simulink model "Scenario_4_power_system_modeling"  (R2023b)
settings: Solver=ode4  StartTime=0.0  StopTime=90  FixedStep=0.01  RelTol=1e-3
values from neighbouring files (symbols this model uses):
  a12 = -1    [simulation_3_Area_scenario.m]    (also in simulation_scenario_1_2_3.m)
  a13 = -1    [simulation_3_Area_scenario.m]
  a23 = -1    [simulation_3_Area_scenario.m]
  b = 0.05    [simulation_3_Area_scenario.m]
  B1 = (1/Rtotal_1) + D1    (= 20.9)    [simulation_3_Area_scenario.m]    (also in simulation_scenario_1_2_3.m = 40.9)
  B2 = (1/Rtotal_2) + D2    (= 21.5)    [simulation_3_Area_scenario.m]    (also in simulation_scenario_1_2_3.m = 41.5)
  B3 = (1/Rtotal_3) + D3    (= 31.3)    [simulation_3_Area_scenario.m]
  c = 1    [simulation_3_Area_scenario.m]
  D1 = 0.9    [simulation_3_Area_scenario.m]    (also in simulation_scenario_1_2_3.m)
  D2 = 1.5    [simulation_3_Area_scenario.m]    (also in simulation_scenario_1_2_3.m)
  D3 = 1.3    [simulation_3_Area_scenario.m]
  Fhp = 0.4    [simulation_3_Area_scenario.m]    (also in simulation_scenario_1_2_3.m)
  Fhp2 = 0.3    [simulation_3_Area_scenario.m]
  Fhp_3 = 0.35    [simulation_3_Area_scenario.m]
  H1 = H1_values(i)    [simulation_3_Area_scenario.m]    (also in simulation_scenario_1_2_3.m)
  H2 = H2_values(i)    [simulation_3_Area_scenario.m]    (also in simulation_scenario_1_2_3.m)
  H3 = H3_values(i)    [simulation_3_Area_scenario.m]
  KI1 = 0.25    [simulation_3_Area_scenario.m]    (also in simulation_scenario_1_2_3.m)
  KI2 = 0.25    [simulation_3_Area_scenario.m]    (also in simulation_scenario_1_2_3.m)
  KI3 = 0.25    [simulation_3_Area_scenario.m]    (also in simulation_scenario_1_2_3.m)
  KI4 = 0.25    [simulation_3_Area_scenario.m]    (also in simulation_scenario_1_2_3.m)
  KI5 = 0.25    [simulation_3_Area_scenario.m]
  KI6 = 0.25    [simulation_3_Area_scenario.m]
  KI7 = 0.25    [simulation_3_Area_scenario.m]
  R1 = 0.1    [simulation_3_Area_scenario.m]    (also in simulation_scenario_1_2_3.m = 0.05)
  R2 = 0.1    [simulation_3_Area_scenario.m]    (also in simulation_scenario_1_2_3.m = 0.05)
  R4 = 0.1    [simulation_3_Area_scenario.m]    (also in simulation_scenario_1_2_3.m = 0.05)
  R5 = 0.1    [simulation_3_Area_scenario.m]
  R6 = 0.1    [simulation_3_Area_scenario.m]
  R7 = 0.1    [simulation_3_Area_scenario.m]
  Rp = 0.05    [simulation_3_Area_scenario.m]    (also in simulation_scenario_1_2_3.m)
  Rt = 0.38    [simulation_3_Area_scenario.m]    (also in simulation_scenario_1_2_3.m)
  T12 = 2    [simulation_3_Area_scenario.m]    (also in simulation_scenario_1_2_3.m)
  T13 = 2    [simulation_3_Area_scenario.m]
  T23 = 2    [simulation_3_Area_scenario.m]
  Tcd = 0.2    [simulation_3_Area_scenario.m]
  Tch1 = 0.39    [simulation_3_Area_scenario.m]    (also in simulation_scenario_1_2_3.m)
  Tch2 = 0.5    [simulation_3_Area_scenario.m]    (also in simulation_scenario_1_2_3.m)
  Tch4 = 0.45    [simulation_3_Area_scenario.m]    (also in simulation_scenario_1_2_3.m)
  Tch5 = 0.4    [simulation_3_Area_scenario.m]
  Tch6 = 0.3    [simulation_3_Area_scenario.m]
  Tcr = 0.01    [simulation_3_Area_scenario.m]
  Tf = 0.23    [simulation_3_Area_scenario.m]
  Tg1 = 0.23    [simulation_3_Area_scenario.m]    (also in simulation_scenario_1_2_3.m)
  Tg2 = 0.14    [simulation_3_Area_scenario.m]    (also in simulation_scenario_1_2_3.m)
  Tg3 = 0.4    [simulation_3_Area_scenario.m]    (also in simulation_scenario_1_2_3.m)
  Tg4 = 0.3    [simulation_3_Area_scenario.m]    (also in simulation_scenario_1_2_3.m)
  Tg5 = 0.1    [simulation_3_Area_scenario.m]
  Tg6 = 0.08    [simulation_3_Area_scenario.m]
  Tr = 5    [simulation_3_Area_scenario.m]    (also in simulation_scenario_1_2_3.m)
  Trh = 7    [simulation_3_Area_scenario.m]    (also in simulation_scenario_1_2_3.m)
  Trh2 = 10    [simulation_3_Area_scenario.m]
  Trh_3 = 5    [simulation_3_Area_scenario.m]
  Tw = 2.25    [simulation_3_Area_scenario.m]    (also in simulation_scenario_1_2_3.m)
  X = 0.6    [simulation_3_Area_scenario.m]
  Y = 1    [simulation_3_Area_scenario.m]
libraries: simulink x4, dspfdesign x3
settings Model: Creator=themi  LastModifiedBy=themi

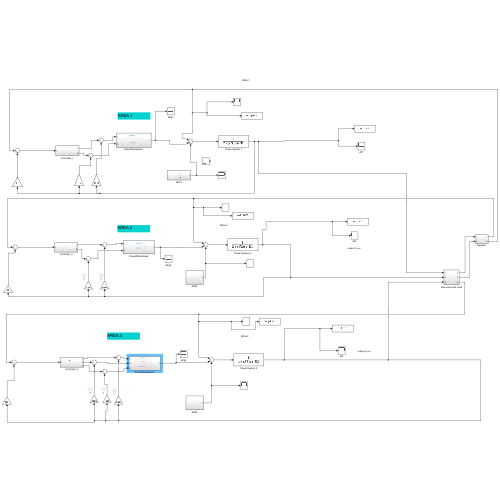
[thumbnail: Simulink's stored preview of the top-level diagram]
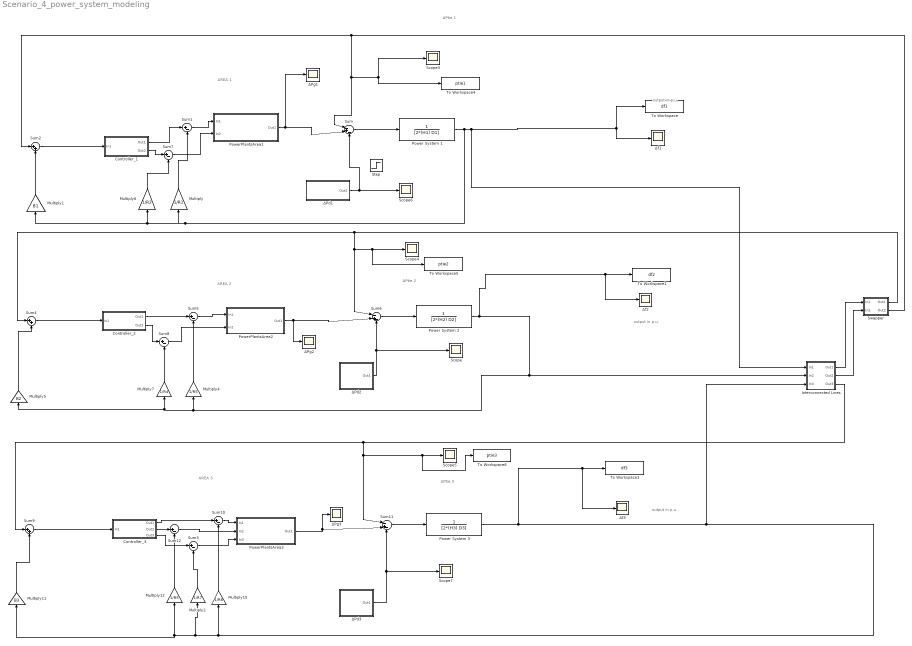
== system Scenario_4_power_system_modeling ==
  note: ΔPtie,1
  note: output in p.u.
  note: output in p.u.
  note: AREA 1
  note: AREA 2
  note: AREA 3
  note: output in p.u.
  note: ΔPtie,2
  note: ΔPtie,3
  Controller_1 : Subsystem
  Controller_2 : Subsystem
  Controller_3 : Subsystem
  Interconnected Lines : Subsystem
  Multiply : Gain  Gain=1/R1
  Multiply1 : Gain  Gain=B1
  Multiply10 : Gain  Gain=1/R6
  Multiply11 : Gain  Gain=B3
  Multiply12 : Gain  Gain=1/R5
  Multiply2 : Gain  Gain=1/R7
  Multiply4 : Gain  Gain=1/R5
  Multiply5 : Gain  Gain=B2
  Multiply6 : Gain  Gain=1/R2
  Multiply7 : Gain  Gain=1/R4
  Power System 1 : TransferFcn  Denominator=[2*(H1) D1]
  Power System 2 : TransferFcn  Denominator=[2*(H2) D2]
  Power System 3 : TransferFcn  Denominator=[2*(H3) D3]
  PowerPlantsArea1 : Subsystem
  PowerPlantsArea2 : Subsystem
  PowerPlantsArea3 : Subsystem
  Scope{,3..7} (6 alike) : Scope
  Step : Step  Time=20  After=0.05  SampleTime=0
  Sum : Sum  Inputs=|-+-
  Sum{1,3,5,7..8,10,12} (7 alike) : Sum  Inputs=|+-
  Sum11 : Sum  Inputs=|-+-
  Sum2 : Sum  Inputs=|++
  Sum4 : Sum  Inputs=|++
  Sum6 : Sum  Inputs=|-+-
  Sum9 : Sum  Inputs=|++
  Swapper : Subsystem
  To Workspace : ToWorkspace  VariableName=df1  SaveFormat=Structure With Time  SampleTime=-1
  To Workspace1 : ToWorkspace  VariableName=df2  SaveFormat=Structure With Time  SampleTime=-1
  To Workspace3 : ToWorkspace  VariableName=df3  SaveFormat=Structure With Time  SampleTime=-1
  To Workspace4 : ToWorkspace  VariableName=ptie1  SaveFormat=Structure With Time  SampleTime=-1
  To Workspace5 : ToWorkspace  VariableName=ptie2  SaveFormat=Structure With Time  SampleTime=-1
  To Workspace6 : ToWorkspace  VariableName=ptie3  SaveFormat=Structure With Time  SampleTime=-1
  ΔPd1 : Subsystem
  ΔPd2 : Subsystem
  ΔPd3 : Subsystem
  ΔPg1 : Scope
  ΔPg2 : Scope
  ΔPg3 : Scope
  Δf1 : Scope
  Δf2 : Scope
  Δf3 : Scope
  wires:
  ΔPd2/1(Out1) -> Scope/1, Sum6/3
  Multiply4/1 -> Sum5/2
  Sum8/1 -> PowerPlantsArea2/2(In2)
  Controller_2/2(Out2) -> Sum8/1
  Sum1/1 -> PowerPlantsArea1/1(In1)
  Sum7/1 -> PowerPlantsArea1/2(In2)
  Power System 2/1 -> Multiply7/1, Multiply5/1, Multiply4/1, Interconnected Lines/2(In2), Δf2/1, To Workspace1/1
  Power System 1/1 -> Interconnected Lines/1(In1), To Workspace/1, Δf1/1, Multiply1/1, Multiply6/1, Multiply/1
  Sum/1 -> Power System 1/1
  Multiply5/1 -> Sum4/2
  Multiply7/1 -> Sum8/2
  Sum4/1 -> Controller_2/1(In1)
  Sum2/1 -> Controller_1/1(In1)
  Controller_2/1(Out1) -> Sum5/1
  PowerPlantsArea2/1(Out1) -> ΔPg2/1, Sum6/2
  PowerPlantsArea1/1(Out1) -> Sum/2, ΔPg1/1
  Sum5/1 -> PowerPlantsArea2/1(In1)
  Sum6/1 -> Power System 2/1
  Multiply12/1 -> Sum12/2
  Power System 3/1 -> Δf3/1, To Workspace3/1, Interconnected Lines/3(In3), Multiply2/1, Multiply12/1, Multiply11/1, Multiply10/1
  Multiply10/1 -> Sum10/2
  Sum12/1 -> PowerPlantsArea3/2(In2)
  Multiply11/1 -> Sum9/2
  Controller_3/2(Out2) -> Sum12/1
  ΔPd3/1(Out1) -> Scope7/1, Sum11/3
  PowerPlantsArea3/1(Out1) -> ΔPg3/1, Sum11/2
  Sum9/1 -> Controller_3/1(In1)
  Sum11/1 -> Power System 3/1
  Controller_3/1(Out1) -> Sum10/1
  Sum10/1 -> PowerPlantsArea3/1(In1)
  Controller_1/1(Out1) -> Sum1/1
  Multiply/1 -> Sum1/2
  Controller_1/2(Out2) -> Sum7/1
  Multiply6/1 -> Sum7/2
  Multiply1/1 -> Sum2/2
  Interconnected Lines/3(Out3) -> Sum11/1, To Workspace6/1, Scope5/1, Sum9/1
  Interconnected Lines/2(Out2) -> Swapper/2(In2)
  Interconnected Lines/1(Out1) -> Swapper/1(In1)
  Swapper/1(Out1) -> To Workspace5/1, Scope4/1, Sum6/1, Sum4/1
  Swapper/2(Out2) -> Sum2/1, Sum/1, Scope3/1, To Workspace4/1
  ΔPd1/1(Out2) -> Scope6/1, Sum/3
  Controller_3/3(Out3) -> Sum3/1
  Multiply2/1 -> Sum3/2
  Sum3/1 -> PowerPlantsArea3/3(In3)

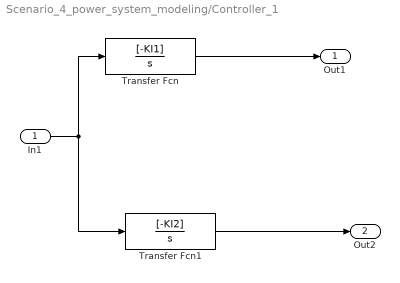
== system Scenario_4_power_system_modeling/Controller_1 ==
  In1 : Inport
  Transfer Fcn : TransferFcn  Numerator=[-KI1]  Denominator=[1 0]
  Transfer Fcn1 : TransferFcn  Numerator=[-KI2]  Denominator=[1 0]
  Out1 : Outport
  Out2 : Outport  Port=2
  wires:
  In1/1 -> Transfer Fcn/1, Transfer Fcn1/1
  Transfer Fcn/1 -> Out1/1
  Transfer Fcn1/1 -> Out2/1

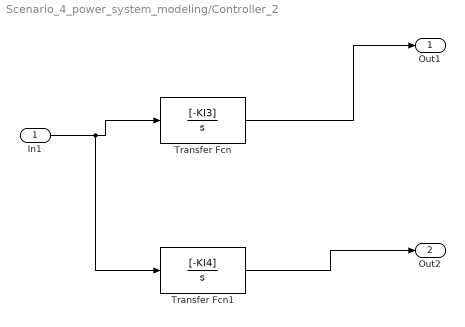
== system Scenario_4_power_system_modeling/Controller_2 ==
  In1 : Inport
  Transfer Fcn : TransferFcn  Numerator=[-KI3]  Denominator=[1 0]
  Transfer Fcn1 : TransferFcn  Numerator=[-KI4]  Denominator=[1 0]
  Out1 : Outport
  Out2 : Outport  Port=2
  wires:
  In1/1 -> Transfer Fcn1/1, Transfer Fcn/1
  Transfer Fcn/1 -> Out1/1
  Transfer Fcn1/1 -> Out2/1

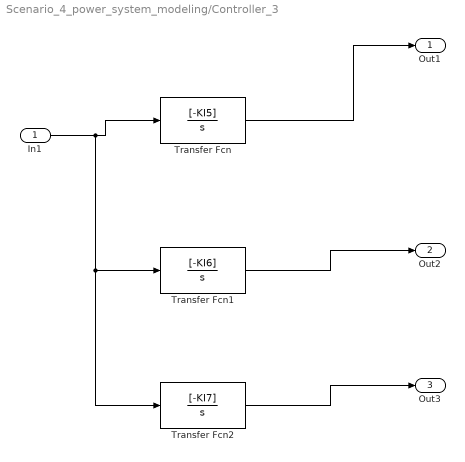
== system Scenario_4_power_system_modeling/Controller_3 ==
  In1 : Inport
  Transfer Fcn : TransferFcn  Numerator=[-KI5]  Denominator=[1 0]
  Transfer Fcn1 : TransferFcn  Numerator=[-KI6]  Denominator=[1 0]
  Transfer Fcn2 : TransferFcn  Numerator=[-KI7]  Denominator=[1 0]
  Out1 : Outport
  Out2 : Outport  Port=2
  Out3 : Outport  Port=3
  wires:
  In1/1 -> Transfer Fcn2/1, Transfer Fcn1/1, Transfer Fcn/1
  Transfer Fcn/1 -> Out1/1
  Transfer Fcn1/1 -> Out2/1
  Transfer Fcn2/1 -> Out3/1

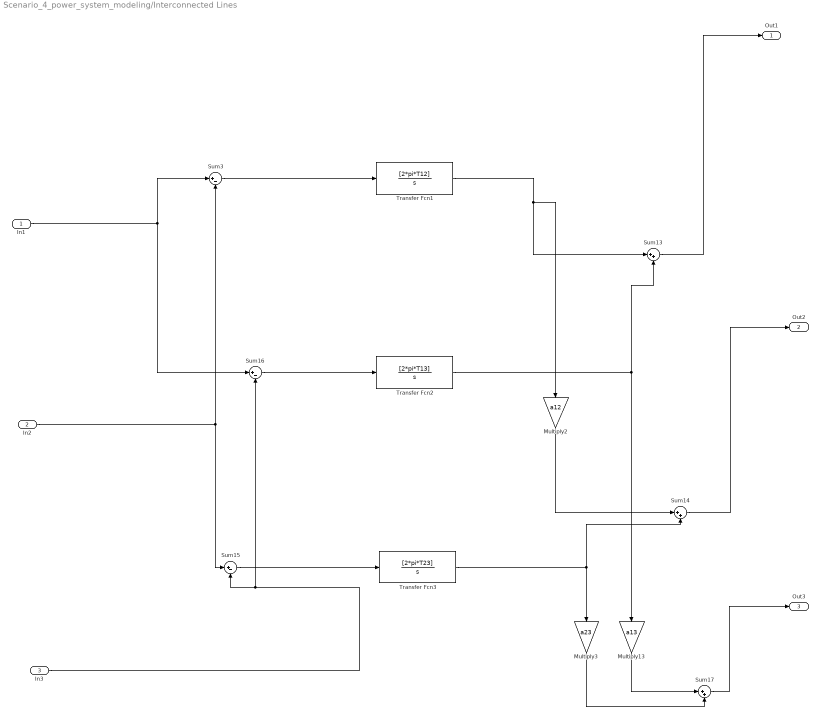
== system Scenario_4_power_system_modeling/Interconnected Lines ==
  In1 : Inport
  In2 : Inport  Port=2
  In3 : Inport  Port=3
  Multiply13 : Gain  Gain=a13
  Multiply2 : Gain  Gain=a12
  Multiply3 : Gain  Gain=a23
  Sum13 : Sum  Inputs=|++
  Sum14 : Sum  Inputs=|++
  Sum15 : Sum  Inputs=|+-
  Sum16 : Sum  Inputs=|+-
  Sum17 : Sum  Inputs=|++
  Sum3 : Sum  Inputs=|+-
  Transfer Fcn1 : TransferFcn  Numerator=[2*pi*T12]  Denominator=[1 0]
  Transfer Fcn2 : TransferFcn  Numerator=[2*pi*T13]  Denominator=[1 0]
  Transfer Fcn3 : TransferFcn  Numerator=[2*pi*T23]  Denominator=[1 0]
  Out1 : Outport
  Out2 : Outport  Port=2
  Out3 : Outport  Port=3
  wires:
  Sum16/1 -> Transfer Fcn2/1
  Transfer Fcn3/1 -> Sum14/2, Multiply3/1
  Transfer Fcn1/1 -> Sum13/1, Multiply2/1
  Transfer Fcn2/1 -> Sum13/2, Multiply13/1
  Multiply2/1 -> Sum14/1
  In3/1 -> Sum16/2, Sum15/2
  In1/1 -> Sum16/1, Sum3/1
  Sum3/1 -> Transfer Fcn1/1
  Multiply13/1 -> Sum17/1
  In2/1 -> Sum15/1, Sum3/2
  Sum15/1 -> Transfer Fcn3/1
  Multiply3/1 -> Sum17/2
  Sum14/1 -> Out2/1
  Sum13/1 -> Out1/1
  Sum17/1 -> Out3/1

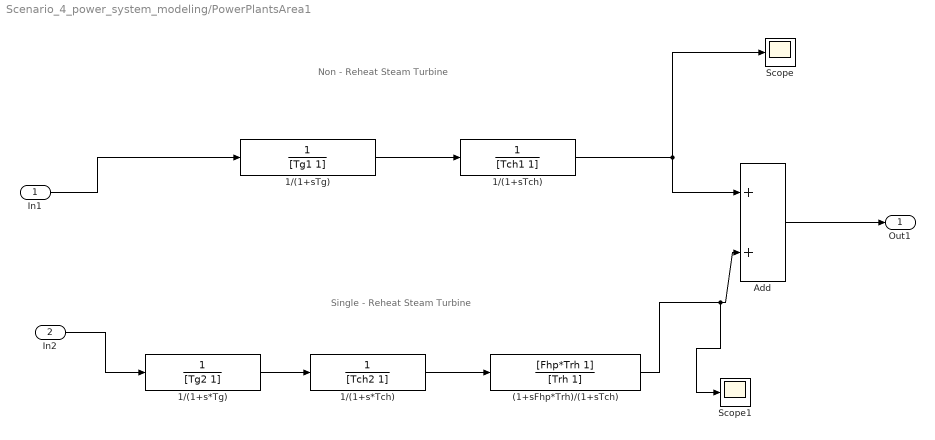
== system Scenario_4_power_system_modeling/PowerPlantsArea1 ==
  note: Non - Reheat Steam Turbine
  note: Single - Reheat Steam Turbine
  In1 : Inport
  In2 : Inport  Port=2
  (1+sFhp*Trh)/(1+sTch) : TransferFcn  Numerator=[Fhp*Trh 1]  Denominator=[Trh 1]
  1/(1+s*Tch) : TransferFcn  Denominator=[Tch2 1]
  1/(1+s*Tg) : TransferFcn  Denominator=[Tg2 1]
  1/(1+sTch) : TransferFcn  Denominator=[Tch1 1]
  1/(1+sTg) : TransferFcn  Denominator=[Tg1 1]
  Add : Sum
  Scope : Scope
  Scope1 : Scope
  Out1 : Outport
  wires:
  In1/1 -> 1/(1+sTg)/1
  1/(1+sTg)/1 -> 1/(1+sTch)/1
  1/(1+s*Tg)/1 -> 1/(1+s*Tch)/1
  1/(1+s*Tch)/1 -> (1+sFhp*Trh)/(1+sTch)/1
  Add/1 -> Out1/1
  1/(1+sTch)/1 -> Scope/1, Add/1
  (1+sFhp*Trh)/(1+sTch)/1 -> Scope1/1, Add/2
  In2/1 -> 1/(1+s*Tg)/1

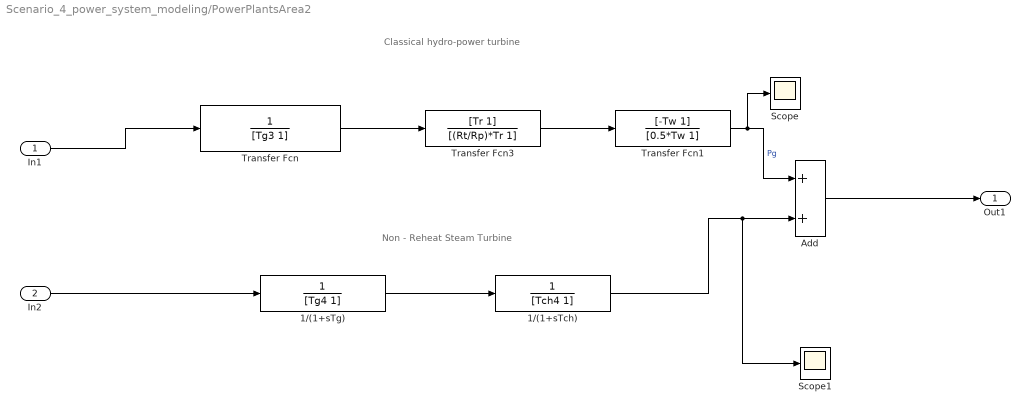
== system Scenario_4_power_system_modeling/PowerPlantsArea2 ==
  note: Classical hydro-power turbine
  note: Non - Reheat Steam Turbine
  In1 : Inport
  In2 : Inport  Port=2
  1/(1+sTch) : TransferFcn  Denominator=[Tch4 1]
  1/(1+sTg) : TransferFcn  Denominator=[Tg4 1]
  Add : Sum
  Scope : Scope
  Scope1 : Scope
  Transfer Fcn : TransferFcn  Denominator=[Tg3 1]
  Transfer Fcn1 : TransferFcn  Numerator=[-Tw 1]  Denominator=[0.5*Tw 1]
  Transfer Fcn3 : TransferFcn  Numerator=[Tr 1]  Denominator=[(Rt/Rp)*Tr 1]
  Out1 : Outport
  wires:
  Transfer Fcn3/1 -> Transfer Fcn1/1
  Transfer Fcn1/1 -> Scope/1, Add/1  "Pg"
  Transfer Fcn/1 -> Transfer Fcn3/1
  In1/1 -> Transfer Fcn/1
  1/(1+sTg)/1 -> 1/(1+sTch)/1
  Add/1 -> Out1/1
  1/(1+sTch)/1 -> Scope1/1, Add/2
  In2/1 -> 1/(1+sTg)/1

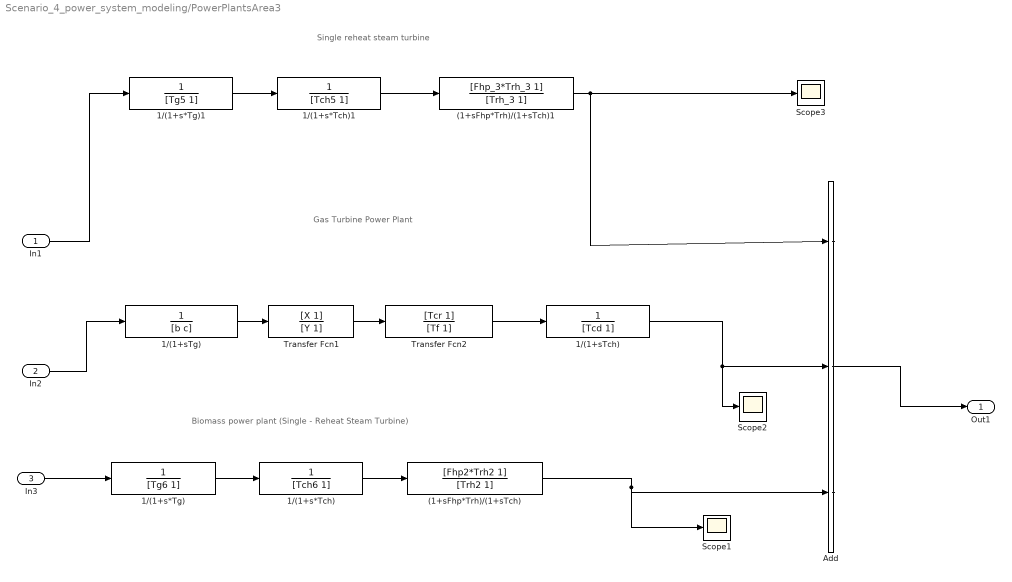
== system Scenario_4_power_system_modeling/PowerPlantsArea3 ==
  note: Gas Turbine Power Plant
  note: Biomass power plant (Single - Reheat Steam Turbine)
  note: Single reheat steam turbine
  In1 : Inport
  In2 : Inport  Port=2
  In3 : Inport  Port=3
  (1+sFhp*Trh)/(1+sTch) : TransferFcn  Numerator=[Fhp2*Trh2 1]  Denominator=[Trh2 1]
  (1+sFhp*Trh)/(1+sTch)1 : TransferFcn  Numerator=[Fhp_3*Trh_3 1]  Denominator=[Trh_3 1]
  1/(1+s*Tch) : TransferFcn  Denominator=[Tch6 1]
  1/(1+s*Tch)1 : TransferFcn  Denominator=[Tch5 1]
  1/(1+s*Tg) : TransferFcn  Denominator=[Tg6 1]
  1/(1+s*Tg)1 : TransferFcn  Denominator=[Tg5 1]
  1/(1+sTch) : TransferFcn  Denominator=[Tcd 1]
  1/(1+sTg) : TransferFcn  Denominator=[b c]
  Add : Sum  Inputs=+++
  Scope1 : Scope
  Scope2 : Scope
  Scope3 : Scope
  Transfer Fcn1 : TransferFcn  Numerator=[X 1]  Denominator=[Y 1]
  Transfer Fcn2 : TransferFcn  Numerator=[Tcr 1]  Denominator=[Tf 1]
  Out1 : Outport
  wires:
  Add/1 -> Out1/1
  1/(1+sTg)/1 -> Transfer Fcn1/1
  Transfer Fcn1/1 -> Transfer Fcn2/1
  Transfer Fcn2/1 -> 1/(1+sTch)/1
  (1+sFhp*Trh)/(1+sTch)/1 -> Scope1/1, Add/3
  In3/1 -> 1/(1+s*Tg)/1
  1/(1+s*Tch)/1 -> (1+sFhp*Trh)/(1+sTch)/1
  1/(1+s*Tg)/1 -> 1/(1+s*Tch)/1
  (1+sFhp*Trh)/(1+sTch)1/1 -> Scope3/1, Add/1
  1/(1+s*Tg)1/1 -> 1/(1+s*Tch)1/1
  1/(1+s*Tch)1/1 -> (1+sFhp*Trh)/(1+sTch)1/1
  In1/1 -> 1/(1+s*Tg)1/1
  In2/1 -> 1/(1+sTg)/1
  1/(1+sTch)/1 -> Scope2/1, Add/2

== system Scenario_4_power_system_modeling/Swapper ==
  In1 : Inport
  In2 : Inport  Port=2
  Out1 : Outport
  Out2 : Outport  Port=2
  wires:
  In1/1 -> Out2/1
  In2/1 -> Out1/1

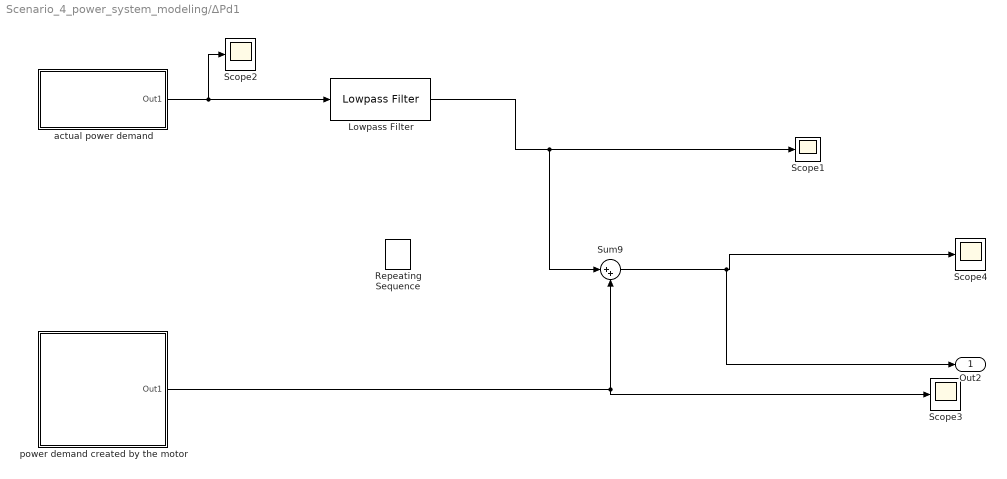
== system Scenario_4_power_system_modeling/ΔPd1 ==
  Lowpass Filter : dspfdesign/Lowpass Filter  FilterType=FIR  DesignForMinimumOrder=on  FilterOrder=50  PassbandFrequency=1  StopbandFrequency=5  PassbandRipple=0.1  StopbandAttenuation=80  SampleRateMode=Specify on dialog  SampleRate=100  SimulateUsing=Interpreted execution  roundingMode=Floor  coeffsDataTypeStr=fixdt(1,16)
  Repeating Sequence : simulink/Sources/Repeating Sequence  rep_seq_t=t  rep_seq_y=disturbance1
  Scope{1..4} (4 alike) : Scope
  Sum9 : Sum  Inputs=|++
  actual power demand : Subsystem
  power demand created by the motor : Subsystem
      mask Display: image('motor.png');
  Out2 : Outport
  wires:
  Sum9/1 -> Out2/1, Scope4/1
  power demand created by the motor/1(Out1) -> Scope3/1, Sum9/2
  actual power demand/1(Out1) -> Scope2/1, Lowpass Filter/1
  Lowpass Filter/1 -> Sum9/1, Scope1/1

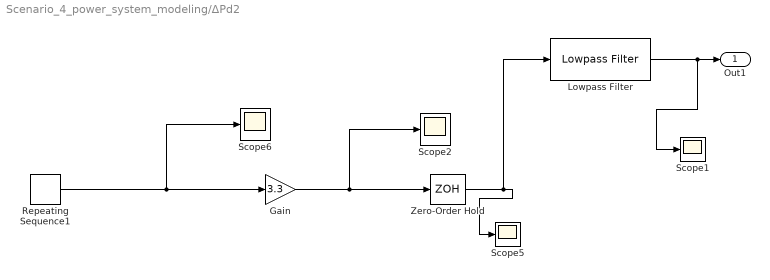
== system Scenario_4_power_system_modeling/ΔPd2 ==
  Gain : Gain  Gain=3.3
  Lowpass Filter : dspfdesign/Lowpass Filter  FilterType=FIR  DesignForMinimumOrder=on  FilterOrder=50  PassbandFrequency=1  StopbandFrequency=5  PassbandRipple=0.1  StopbandAttenuation=80  SampleRateMode=Specify on dialog  SampleRate=100  SimulateUsing=Interpreted execution  roundingMode=Floor  coeffsDataTypeStr=fixdt(1,16)
  Repeating Sequence1 : simulink/Sources/Repeating Sequence  rep_seq_t=t  rep_seq_y=disturbance2
  Scope{1..2,5..6} (4 alike) : Scope
  Zero-Order Hold : ZeroOrderHold  SampleTime=0.01
  Out1 : Outport
  wires:
  Gain/1 -> Scope2/1, Zero-Order Hold/1
  Zero-Order Hold/1 -> Lowpass Filter/1, Scope5/1
  Repeating Sequence1/1 -> Scope6/1, Gain/1
  Lowpass Filter/1 -> Scope1/1, Out1/1

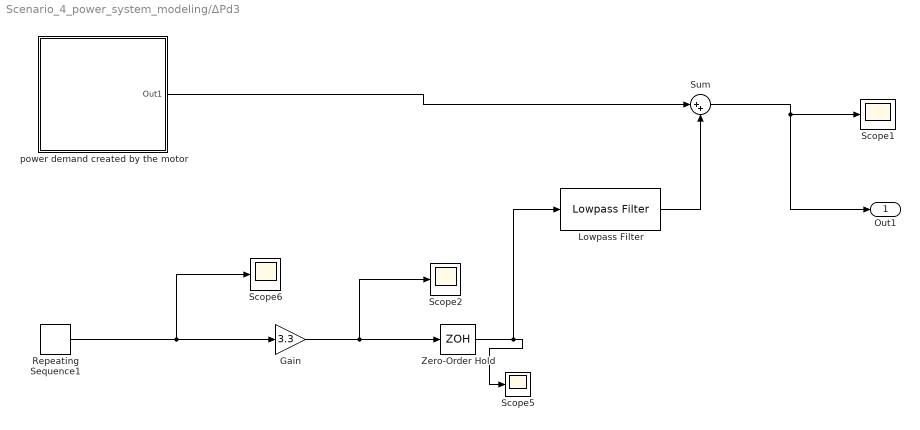
== system Scenario_4_power_system_modeling/ΔPd3 ==
  Gain : Gain  Gain=3.3
  Lowpass Filter : dspfdesign/Lowpass Filter  FilterType=FIR  DesignForMinimumOrder=on  FilterOrder=50  PassbandFrequency=1  StopbandFrequency=5  PassbandRipple=0.1  StopbandAttenuation=80  SampleRateMode=Specify on dialog  SampleRate=100  SimulateUsing=Interpreted execution  roundingMode=Floor  coeffsDataTypeStr=fixdt(1,16)
  Repeating Sequence1 : simulink/Sources/Repeating Sequence  rep_seq_t=t  rep_seq_y=disturbance3
  Scope{1..2,5..6} (4 alike) : Scope
  Sum : Sum  Inputs=|++
  Zero-Order Hold : ZeroOrderHold  SampleTime=0.01
  power demand created by the motor : Subsystem
      mask Display: image('motor.png');
  Out1 : Outport
  wires:
  Gain/1 -> Scope2/1, Zero-Order Hold/1
  Zero-Order Hold/1 -> Lowpass Filter/1, Scope5/1
  Repeating Sequence1/1 -> Scope6/1, Gain/1
  Lowpass Filter/1 -> Sum/2
  power demand created by the motor/1(Out1) -> Sum/1
  Sum/1 -> Out1/1, Scope1/1

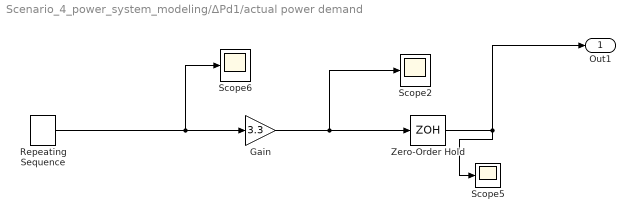
== system Scenario_4_power_system_modeling/ΔPd1/actual power demand ==
  Gain : Gain  Gain=3.3
  Repeating Sequence : simulink/Sources/Repeating Sequence  rep_seq_t=t  rep_seq_y=disturbance1
  Scope2 : Scope
  Scope5 : Scope
  Scope6 : Scope
  Zero-Order Hold : ZeroOrderHold  SampleTime=0.01
  Out1 : Outport
  wires:
  Gain/1 -> Scope2/1, Zero-Order Hold/1
  Repeating Sequence/1 -> Scope6/1, Gain/1
  Zero-Order Hold/1 -> Out1/1, Scope5/1

== system Scenario_4_power_system_modeling/ΔPd1/power demand created by the motor ==
  note: ΔPd1(s)
  Step1 : Step  Time=20  After=0.01  SampleTime=0
  Step2 : Step  Time=40  After=0.01  SampleTime=0
  Sum10 : Sum  Inputs=|+-
  Out1 : Outport
  wires:
  Step2/1 -> Sum10/2
  Step1/1 -> Sum10/1
  Sum10/1 -> Out1/1

== system Scenario_4_power_system_modeling/ΔPd3/power demand created by the motor ==
  Step1 : Step  Time=20  After=0.01  SampleTime=0
  Step2 : Step  Time=40  After=0.01  SampleTime=0
  Sum10 : Sum  Inputs=|+-
  Out1 : Outport
  wires:
  Step2/1 -> Sum10/2
  Step1/1 -> Sum10/1
  Sum10/1 -> Out1/1
